simulink model "nb_107_32k_nb_fft_only_vlbi"  (R2018a) (continued)
== system nb_107_32k_nb_fft_only_vlbi == (continued)
      | port_label('input', 1, 'd');
      | port_label('output', 1, 'q');
      | disp(['Z^-', num2str(latency)]);
  pipeline22 : Subsystem  AncestorBlock=casper_library_delays/pipeline  mask(pipeline){latency=2}
      mask help:
      | An explicitly laid-out delay line to avoid mapping to SRL16s
      | for use in pipelining to help achieve timing closure.
      mask init:
      | pipeline_init(gcb,'latency',latency);
      settings: AttributesFormatString=Latency = %<latency>
      mask Display:
      | port_label('input', 1, 'd');
      | port_label('output', 1, 'q');
      | disp(['Z^-', num2str(latency)]);
  pipeline223 : Subsystem  Commented=on  AncestorBlock=casper_library_delays/pipeline  mask(pipeline){latency=5}
      mask help:
      | An explicitly laid-out delay line to avoid mapping to SRL16s
      | for use in pipelining to help achieve timing closure.
      mask init:
      | pipeline_init(gcb,'latency',latency);
      settings: AttributesFormatString=Latency = %<latency>
      mask Display:
      | port_label('input', 1, 'd');
      | port_label('output', 1, 'q');
      | disp(['Z^-', num2str(latency)]);
  pipeline23 : Subsystem  AncestorBlock=casper_library_delays/pipeline  mask(pipeline){csp_latency=2}
      mask prompts: csp_latency "Latency"
      mask help:
      | An explicitly laid-out delay line to avoid mapping to SRL16s
      | for use in pipelining to help achieve timing closure.
      mask init:
      | pipeline_init(gcb,'latency',csp_latency);
      settings: AttributesFormatString=Latency = %<latency>
      mask Display:
      | port_label('input', 1, 'd');
      | port_label('output', 1, 'q');
      | disp(['Z^-', num2str(csp_latency)]);
  pipeline234 : Subsystem  Commented=on  AncestorBlock=casper_library_delays/pipeline  mask(pipeline){latency=2}
      mask help:
      | An explicitly laid-out delay line to avoid mapping to SRL16s
      | for use in pipelining to help achieve timing closure.
      mask init:
      | pipeline_init(gcb,'latency',latency);
      settings: AttributesFormatString=Latency = %<latency>
      mask Display:
      | port_label('input', 1, 'd');
      | port_label('output', 1, 'q');
      | disp(['Z^-', num2str(latency)]);
  pipeline24 : Subsystem  AncestorBlock=casper_library_delays/pipeline  mask(pipeline){csp_latency=2}
      mask prompts: csp_latency "Latency"
      mask help:
      | An explicitly laid-out delay line to avoid mapping to SRL16s
      | for use in pipelining to help achieve timing closure.
      mask init:
      | pipeline_init(gcb,'latency',csp_latency);
      settings: AttributesFormatString=Latency = %<latency>
      mask Display:
      | port_label('input', 1, 'd');
      | port_label('output', 1, 'q');
      | disp(['Z^-', num2str(csp_latency)]);
  pipeline25 : Subsystem  AncestorBlock=casper_library_delays/pipeline  mask(pipeline){csp_latency=2}
      mask prompts: csp_latency "Latency"
      mask help:
      | An explicitly laid-out delay line to avoid mapping to SRL16s
      | for use in pipelining to help achieve timing closure.
      mask init:
      | pipeline_init(gcb,'latency',csp_latency);
      settings: AttributesFormatString=Latency = %<latency>
      mask Display:
      | port_label('input', 1, 'd');
      | port_label('output', 1, 'q');
      | disp(['Z^-', num2str(csp_latency)]);
  pipeline26 : Subsystem  AncestorBlock=casper_library_delays/pipeline  mask(pipeline){csp_latency=2}
      mask prompts: csp_latency "Latency"
      mask help:
      | An explicitly laid-out delay line to avoid mapping to SRL16s
      | for use in pipelining to help achieve timing closure.
      mask init:
      | pipeline_init(gcb,'latency',csp_latency);
      settings: AttributesFormatString=Latency = %<latency>
      mask Display:
      | port_label('input', 1, 'd');
      | port_label('output', 1, 'q');
      | disp(['Z^-', num2str(csp_latency)]);
  pipeline27 : Subsystem  AncestorBlock=casper_library_delays/pipeline  mask(pipeline){csp_latency=2}
      mask prompts: csp_latency "Latency"
      mask help:
      | An explicitly laid-out delay line to avoid mapping to SRL16s
      | for use in pipelining to help achieve timing closure.
      mask init:
      | pipeline_init(gcb,'latency',csp_latency);
      settings: AttributesFormatString=Latency = %<latency>
      mask Display:
      | port_label('input', 1, 'd');
      | port_label('output', 1, 'q');
      | disp(['Z^-', num2str(csp_latency)]);
  pipeline28 : Subsystem  AncestorBlock=casper_library_delays/pipeline  mask(pipeline){csp_latency=2}
      mask prompts: csp_latency "Latency"
      mask help:
      | An explicitly laid-out delay line to avoid mapping to SRL16s
      | for use in pipelining to help achieve timing closure.
      mask init:
      | pipeline_init(gcb,'latency',csp_latency);
      settings: AttributesFormatString=Latency = %<latency>
      mask Display:
      | port_label('input', 1, 'd');
      | port_label('output', 1, 'q');
      | disp(['Z^-', num2str(csp_latency)]);
  pipeline31 : Subsystem  AncestorBlock=casper_library_delays/pipeline  mask(pipeline){latency=2}
      mask help:
      | An explicitly laid-out delay line to avoid mapping to SRL16s
      | for use in pipelining to help achieve timing closure.
      mask init:
      | pipeline_init(gcb,'latency',latency);
      settings: AttributesFormatString=Latency = %<latency>
      mask Display:
      | port_label('input', 1, 'd');
      | port_label('output', 1, 'q');
      | disp(['Z^-', num2str(latency)]);
  pipeline38 : Subsystem  AncestorBlock=casper_library_delays/pipeline  mask(pipeline){csp_latency=2}
      mask prompts: csp_latency "Latency"
      mask help:
      | An explicitly laid-out delay line to avoid mapping to SRL16s
      | for use in pipelining to help achieve timing closure.
      mask init:
      | pipeline_init(gcb,'latency',csp_latency);
      settings: AttributesFormatString=Latency = %<latency>
      mask Display:
      | port_label('input', 1, 'd');
      | port_label('output', 1, 'q');
      | disp(['Z^-', num2str(csp_latency)]);
  pipeline39 : Subsystem  AncestorBlock=casper_library_delays/pipeline  mask(pipeline){csp_latency=2}
      mask prompts: csp_latency "Latency"
      mask help:
      | An explicitly laid-out delay line to avoid mapping to SRL16s
      | for use in pipelining to help achieve timing closure.
      mask init:
      | pipeline_init(gcb,'latency',csp_latency);
      settings: AttributesFormatString=Latency = %<latency>
      mask Display:
      | port_label('input', 1, 'd');
      | port_label('output', 1, 'q');
      | disp(['Z^-', num2str(csp_latency)]);
  pipeline4 : Subsystem  Commented=on  AncestorBlock=casper_library_delays/pipeline  mask(pipeline){latency=5}
      mask help:
      | An explicitly laid-out delay line to avoid mapping to SRL16s
      | for use in pipelining to help achieve timing closure.
      mask init:
      | pipeline_init(gcb,'latency',latency);
      settings: AttributesFormatString=Latency = %<latency>
      mask Display:
      | port_label('input', 1, 'd');
      | port_label('output', 1, 'q');
      | disp(['Z^-', num2str(latency)]);
  pipeline43 : Subsystem  AncestorBlock=casper_library_delays/pipeline  mask(pipeline){csp_latency=2}
      mask prompts: csp_latency "Latency"
      mask help:
      | An explicitly laid-out delay line to avoid mapping to SRL16s
      | for use in pipelining to help achieve timing closure.
      mask init:
      | pipeline_init(gcb,'latency',csp_latency);
      settings: AttributesFormatString=Latency = %<latency>
      mask Display:
      | port_label('input', 1, 'd');
      | port_label('output', 1, 'q');
      | disp(['Z^-', num2str(csp_latency)]);
  pipeline46 : Subsystem  Tag=cmac_delinked  mask(pipeline){csp_latency=2}
      mask prompts: csp_latency "Latency"
      mask help:
      | An explicitly laid-out delay line to avoid mapping to SRL16s
      | for use in pipelining to help achieve timing closure.
      mask init:
      | pipeline_init(gcb,'latency',csp_latency);
      settings: AttributesFormatString=Latency = %<latency>
      mask Display:
      | port_label('input', 1, 'd');
      | port_label('output', 1, 'q');
      | disp(['Z^-', num2str(csp_latency)]);
  pipeline47 : Subsystem  AncestorBlock=casper_library_delays/pipeline  mask(pipeline){csp_latency=2}
      mask prompts: csp_latency "Latency"
      mask help:
      | An explicitly laid-out delay line to avoid mapping to SRL16s
      | for use in pipelining to help achieve timing closure.
      mask init:
      | pipeline_init(gcb,'latency',csp_latency);
      settings: AttributesFormatString=Latency = %<latency>
      mask Display:
      | port_label('input', 1, 'd');
      | port_label('output', 1, 'q');
      | disp(['Z^-', num2str(csp_latency)]);
  pipeline5 : Subsystem  Commented=on  AncestorBlock=casper_library_delays/pipeline  mask(pipeline){latency=5}
      mask help:
      | An explicitly laid-out delay line to avoid mapping to SRL16s
      | for use in pipelining to help achieve timing closure.
      mask init:
      | pipeline_init(gcb,'latency',latency);
      settings: AttributesFormatString=Latency = %<latency>
      mask Display:
      | port_label('input', 1, 'd');
      | port_label('output', 1, 'q');
      | disp(['Z^-', num2str(latency)]);
  pipeline51 : Subsystem  AncestorBlock=casper_library_delays/pipeline  mask(pipeline){latency=2}
      mask help:
      | An explicitly laid-out delay line to avoid mapping to SRL16s
      | for use in pipelining to help achieve timing closure.
      mask init:
      | pipeline_init(gcb,'latency',latency);
      settings: AttributesFormatString=Latency = %<latency>
      mask Display:
      | port_label('input', 1, 'd');
      | port_label('output', 1, 'q');
      | disp(['Z^-', num2str(latency)]);
  pipeline52 : Subsystem  AncestorBlock=casper_library_delays/pipeline  mask(pipeline){csp_latency=2}
      mask prompts: csp_latency "Latency"
      mask help:
      | An explicitly laid-out delay line to avoid mapping to SRL16s
      | for use in pipelining to help achieve timing closure.
      mask init:
      | pipeline_init(gcb,'latency',csp_latency);
      settings: AttributesFormatString=Latency = %<latency>
      mask Display:
      | port_label('input', 1, 'd');
      | port_label('output', 1, 'q');
      | disp(['Z^-', num2str(csp_latency)]);
  pipeline59 : Subsystem  AncestorBlock=casper_library_delays/pipeline  mask(pipeline){csp_latency=2}
      mask prompts: csp_latency "Latency"
      mask help:
      | An explicitly laid-out delay line to avoid mapping to SRL16s
      | for use in pipelining to help achieve timing closure.
      mask init:
      | pipeline_init(gcb,'latency',csp_latency);
      settings: AttributesFormatString=Latency = %<latency>
      mask Display:
      | port_label('input', 1, 'd');
      | port_label('output', 1, 'q');
      | disp(['Z^-', num2str(csp_latency)]);
  pipeline6 : Subsystem  Commented=on  AncestorBlock=casper_library_delays/pipeline  mask(pipeline){latency=5}
      mask help:
      | An explicitly laid-out delay line to avoid mapping to SRL16s
      | for use in pipelining to help achieve timing closure.
      mask init:
      | pipeline_init(gcb,'latency',latency);
      settings: AttributesFormatString=Latency = %<latency>
      mask Display:
      | port_label('input', 1, 'd');
      | port_label('output', 1, 'q');
      | disp(['Z^-', num2str(latency)]);
  pipeline62 : Subsystem  AncestorBlock=casper_library_delays/pipeline  mask(pipeline){latency=2}
      mask help:
      | An explicitly laid-out delay line to avoid mapping to SRL16s
      | for use in pipelining to help achieve timing closure.
      mask init:
      | pipeline_init(gcb,'latency',latency);
      settings: AttributesFormatString=Latency = %<latency>
      mask Display:
      | port_label('input', 1, 'd');
      | port_label('output', 1, 'q');
      | disp(['Z^-', num2str(latency)]);
  pipeline66 : Subsystem  AncestorBlock=casper_library_delays/pipeline  mask(pipeline){csp_latency=2}
      mask prompts: csp_latency "Latency"
      mask help:
      | An explicitly laid-out delay line to avoid mapping to SRL16s
      | for use in pipelining to help achieve timing closure.
      mask init:
      | pipeline_init(gcb,'latency',csp_latency);
      settings: AttributesFormatString=Latency = %<latency>
      mask Display:
      | port_label('input', 1, 'd');
      | port_label('output', 1, 'q');
      | disp(['Z^-', num2str(csp_latency)]);
  pipeline7 : Subsystem  Commented=on  AncestorBlock=casper_library_delays/pipeline  mask(pipeline){latency=5}
      mask help:
      | An explicitly laid-out delay line to avoid mapping to SRL16s
      | for use in pipelining to help achieve timing closure.
      mask init:
      | pipeline_init(gcb,'latency',latency);
      settings: AttributesFormatString=Latency = %<latency>
      mask Display:
      | port_label('input', 1, 'd');
      | port_label('output', 1, 'q');
      | disp(['Z^-', num2str(latency)]);
  pipeline8 : Subsystem  AncestorBlock=casper_library_delays/pipeline  mask(pipeline){csp_latency=2}
      mask prompts: csp_latency "Latency"
      mask help:
      | An explicitly laid-out delay line to avoid mapping to SRL16s
      | for use in pipelining to help achieve timing closure.
      mask init:
      | pipeline_init(gcb,'latency',csp_latency);
      settings: AttributesFormatString=Latency = %<latency>
      mask Display:
      | port_label('input', 1, 'd');
      | port_label('output', 1, 'q');
      | disp(['Z^-', num2str(csp_latency)]);
  pipeline9 : Subsystem  Commented=on  AncestorBlock=casper_library_delays/pipeline  mask(pipeline){latency=5}
      mask help:
      | An explicitly laid-out delay line to avoid mapping to SRL16s
      | for use in pipelining to help achieve timing closure.
      mask init:
      | pipeline_init(gcb,'latency',latency);
      settings: AttributesFormatString=Latency = %<latency>
      mask Display:
      | port_label('input', 1, 'd');
      | port_label('output', 1, 'q');
      | disp(['Z^-', num2str(latency)]);
  pipeline93 : Subsystem  AncestorBlock=casper_library_delays/pipeline  mask(pipeline){csp_latency=2}
      mask prompts: csp_latency "Latency"
      mask help:
      | An explicitly laid-out delay line to avoid mapping to SRL16s
      | for use in pipelining to help achieve timing closure.
      mask init:
      | pipeline_init(gcb,'latency',csp_latency);
      settings: AttributesFormatString=Latency = %<latency>
      mask Display:
      | port_label('input', 1, 'd');
      | port_label('output', 1, 'q');
      | disp(['Z^-', num2str(csp_latency)]);
  pipeline96 : Subsystem  AncestorBlock=casper_library_delays/pipeline  mask(pipeline){latency=2}
      mask help:
      | An explicitly laid-out delay line to avoid mapping to SRL16s
      | for use in pipelining to help achieve timing closure.
      mask init:
      | pipeline_init(gcb,'latency',latency);
      settings: AttributesFormatString=Latency = %<latency>
      mask Display:
      | port_label('input', 1, 'd');
      | port_label('output', 1, 'q');
      | disp(['Z^-', num2str(latency)]);
  pipeline97 : Subsystem  AncestorBlock=casper_library_delays/pipeline  mask(pipeline){latency=2}
      mask help:
      | An explicitly laid-out delay line to avoid mapping to SRL16s
      | for use in pipelining to help achieve timing closure.
      mask init:
      | pipeline_init(gcb,'latency',latency);
      settings: AttributesFormatString=Latency = %<latency>
      mask Display:
      | port_label('input', 1, 'd');
      | port_label('output', 1, 'q');
      | disp(['Z^-', num2str(latency)]);
  pipeline98 : Subsystem  AncestorBlock=casper_library_delays/pipeline  mask(pipeline){latency=2}
      mask help:
      | An explicitly laid-out delay line to avoid mapping to SRL16s
      | for use in pipelining to help achieve timing closure.
      mask init:
      | pipeline_init(gcb,'latency',latency);
      settings: AttributesFormatString=Latency = %<latency>
      mask Display:
      | port_label('input', 1, 'd');
      | port_label('output', 1, 'q');
      | disp(['Z^-', num2str(latency)]);
  pipeline99 : Subsystem  AncestorBlock=casper_library_delays/pipeline  mask(pipeline){latency=2}
      mask help:
      | An explicitly laid-out delay line to avoid mapping to SRL16s
      | for use in pipelining to help achieve timing closure.
      mask init:
      | pipeline_init(gcb,'latency',latency);
      settings: AttributesFormatString=Latency = %<latency>
      mask Display:
      | port_label('input', 1, 'd');
      | port_label('output', 1, 'q');
      | disp(['Z^-', num2str(latency)]);
  pkt_len : Subsystem  Tag=xps:sw_reg  AncestorBlock=xps_library/Memory/software_register  mask(swreg){io_dir=From Processor, io_delay=1, init_val=2^9-1, sample_period=1, names=len, bitwidths=9, bin_pts=0, arith_types=0, sim_port=on, show_format=on}
      mask prompts: io_dir "I/O direction"; io_delay "I/O delay"; init_val "Initial Value"; sample_period "Sample period"; names "Bitfield names [msb...lsb]"; bitwidths "Bitfield widths"; bin_pts "Bitfield binary pts"; arith_types "Bitfield types, ufix=0, fix=1, bool=2"; sim_port "Provide sim input/output?"; show_format "Print format string?"
      mask help: A 32-bit software-accessible register. Can be divided into bitfields of varying widths and types using the fields in the block mask.
      mask init:
      | swreg_init(gcb)
      settings: AttributesFormatString=1input:uf9.0 = 9 bits
      mask TypeOptions io_dir: To Processor | From Processor
      mask Callback io_dir:
      | mask_enables = get_param(gcb, 'MaskEnables');
      | mask_names = get_param(gcb, 'MaskNames');
      | if strcmp(get_param(gcb, 'io_dir'), 'To Processor'),
      |     mask_enables{ismember(mask_names, 'sample_period')} = 'off';
      | else
      |     mask_enables{ismember(mask_names, 'sample_period')} = 'on';
      | end
      | set_param(gcb, 'MaskEnables', mask_enables);
      mask DialogControl ParameterTabVar0 Prompt: Setup
  pkt_len_sel : Subsystem  Tag=xps:sw_reg  AncestorBlock=xps_library/Memory/software_register  mask(swreg){io_dir=From Processor, io_delay=1, init_val=0, sample_period=1, names=sel, bitwidths=1, bin_pts=0, arith_types=2, sim_port=on, show_format=on}
      mask prompts: io_dir "I/O direction"; io_delay "I/O delay"; init_val "Initial Value"; sample_period "Sample period"; names "Bitfield names [msb...lsb]"; bitwidths "Bitfield widths"; bin_pts "Bitfield binary pts"; arith_types "Bitfield types, ufix=0, fix=1, bool=2"; sim_port "Provide sim input/output?"; show_format "Print format string?"
      mask help: A 32-bit software-accessible register. Can be divided into bitfields of varying widths and types using the fields in the block mask.
      mask init:
      | swreg_init(gcb)
      settings: AttributesFormatString=1input:b = 1 bits
      mask TypeOptions io_dir: To Processor | From Processor
      mask Callback io_dir:
      | mask_enables = get_param(gcb, 'MaskEnables');
      | mask_names = get_param(gcb, 'MaskNames');
      | if strcmp(get_param(gcb, 'io_dir'), 'To Processor'),
      |     mask_enables{ismember(mask_names, 'sample_period')} = 'off';
      | else
      |     mask_enables{ismember(mask_names, 'sample_period')} = 'on';
      | end
      | set_param(gcb, 'MaskEnables', mask_enables);
      mask DialogControl ParameterTabVar0 Prompt: Setup
  re_im_interleave : Subsystem
  rx_dest_ip : Subsystem  Tag=xps:sw_reg  AncestorBlock=xps_library/software register  mask(swreg){io_dir=To Processor, io_delay=0, sample_period=1, names=reg, bitwidths=32, bin_pts=0, arith_types=0, sim_port=off, show_format=on}
      mask prompts: io_dir "I/O direction"; io_delay "I/O delay"; sample_period "Sample period"; names "Bitfield names [msb...lsb]"; bitwidths "Bitfield widths"; bin_pts "Bitfield binary pts"; arith_types "Bitfield types, ufix=0, fix=1, bool=2"; sim_port "Provide sim input/output?"; show_format "Print format string?"
      mask help: A 32-bit software-accessible register. Can be divided into bitfields of varying widths and types using the fields in the block mask.
      mask init:
      | swreg_init(gcb)
      settings: AttributesFormatString=1output:uf32.0 = 32 bits
      mask TypeOptions io_dir: To Processor | From Processor
      mask Callback io_dir:
      | mask_enables = get_param(gcb, 'MaskEnables');
      | mask_names = get_param(gcb, 'MaskNames');
      | if strcmp(get_param(gcb, 'io_dir'), 'To Processor'),
      |     mask_enables{ismember(mask_names, 'sample_period')} = 'off';
      | else
      |     mask_enables{ismember(mask_names, 'sample_period')} = 'on';
      | end
      | set_param(gcb, 'MaskEnables', mask_enables);
  scale_wng0 : Subsystem  Tag=xps:sw_reg  AncestorBlock=xps_library/Memory/software_register  mask(swreg){io_dir=From Processor, io_delay=1, init_val=0, sample_period=1, names=scale, bitwidths=16, bin_pts=15, arith_types=0, sim_port=on, show_format=on}
      mask prompts: io_dir "I/O direction"; io_delay "I/O delay"; init_val "Initial Value"; sample_period "Sample period"; names "Bitfield names [msb...lsb]"; bitwidths "Bitfield widths"; bin_pts "Bitfield binary pts"; arith_types "Bitfield types, ufix=0, fix=1, bool=2"; sim_port "Provide sim input/output?"; show_format "Print format string?"
      mask help: A 32-bit software-accessible register. Can be divided into bitfields of varying widths and types using the fields in the block mask.
      mask init:
      | swreg_init(gcb)
      settings: AttributesFormatString=1input:uf16.15 = 16 bits
      mask TypeOptions io_dir: To Processor | From Processor
      mask Callback io_dir:
      | mask_enables = get_param(gcb, 'MaskEnables');
      | mask_names = get_param(gcb, 'MaskNames');
      | if strcmp(get_param(gcb, 'io_dir'), 'To Processor'),
      |     mask_enables{ismember(mask_names, 'sample_period')} = 'off';
      | else
      |     mask_enables{ismember(mask_names, 'sample_period')} = 'on';
      | end
      | set_param(gcb, 'MaskEnables', mask_enables);
      mask DialogControl ParameterTabVar0 Prompt: Setup
  snap_cwg : Subsystem  Tag=casper:bitsnap  Commented=on  AncestorBlock=casper_library_scopes/bitfield_snapshot  mask(bitsnap){io_names=[p0_d0 p0_d1 p0_d2 p0_d3 p0_d4 p0_d5 p0_d6 p0_d7 dv sync], io_widths=[10 10 10 10 10 10 10 10 1 1], io_bps=[9 9 9 9 9 9 9 9 0 0], io_types=[1 1 1 1 1 1 1 1 2 2], show_format=on, snap_storage=bram, snap_dram_dimm=2, snap_dram_clock=250, snap_nsamples=10, snap_data_width=128, snap_offset=off, snap_circap=off, snap_value=off, snap_ext_arm=off, snap_ext_circ=off, snap_provide_outputs=on, snap_use_dsp48=on, snap_delay=2, extra_names=timestamp, extra_widths=32, extra_bps=0, extra_types=0}
      mask prompts: io_names "Names [msb...lsb]"; io_widths "Bitwidths [msb...lsb]"; io_bps "Binary pts [msb...lsb]"; io_types "Data types [msb...lsb], ufix=0, fix=1, bool=2"; show_format "Print format string?"; snap_storage "Storage medium"; snap_dram_dimm "DRAM dimm"; snap_dram_clock "DRAM clock rate"; snap_nsamples "Number of Samples (2^?)"; snap_data_width "Data width"; snap_offset "Start delay support"; snap_circap "Circular capture support"; snap_value "Extra value capture support"; snap_ext_arm "External arm support"; snap_ext_circ "External circular capture support"; snap_provide_outputs "Provide outputs"; snap_use_dsp48 "Use DSP48s to implement counters"; snap_delay "Pre-snapshot delay"; extra_names "Names [msb...lsb]"; extra_widths "Widths [msb...lsb]"; extra_bps "Binary pts [msb...lsb]"; extra_types "Data types [msb...lsb], ufix=0, fix=1, bool=2"
      mask help:
      | A wrapped snapshot block. Allows specification of inputs to a snapblock word so the toolflow can automatically set up the scope.
      | See documentation for snapshot for explanation of parameters.
      mask init:
      | bitsnap_init(gcb);
      settings: AttributesFormatString=|
      | snap(10):f10.9,f10.9,f10.9,f10.9,f10.9,f10.9,f10.9,f10.9,b,b = 82 bits\n128 wide, 1024 deep
      | debugID: 22238404
      mask TypeOptions snap_storage: bram | dram
      mask Callback snap_storage: bitsnap_callback();
      mask TypeOptions snap_dram_dimm: 1 | 2 | 3 | 4
      mask TypeOptions snap_dram_clock: 200 | 250 | 266 | 300 | 333
      mask TypeOptions snap_data_width: 8 | 16 | 32 | 64 | 128
      mask Callback snap_value: bitsnap_callback();
      mask Callback snap_ext_arm: bitsnap_callback();
      mask Callback snap_ext_circ: bitsnap_callback();
      mask Callback snap_provide_outputs: bitsnap_callback();
      mask DialogControl ParameterTabVar0 Prompt: Inputs
      mask DialogControl ParameterTabVar01 Prompt: Snapshot
      mask DialogControl ParameterTabVar012 Prompt: Extra Value
  snap_dds : Subsystem  Tag=casper:bitsnap  Commented=on  AncestorBlock=casper_library_scopes/bitfield_snapshot  mask(bitsnap){io_names=[p0_d0 p0_d1 p0_d2 p0_d3 dv sync], io_widths=[18 18 18 18 1 1], io_bps=[17 17 17 17 0 0], io_types=[1 1 1 1 2 2], show_format=on, snap_storage=bram, snap_dram_dimm=2, snap_dram_clock=250, snap_nsamples=10, snap_data_width=128, snap_offset=off, snap_circap=off, snap_value=off, snap_ext_arm=off, snap_ext_circ=off, snap_provide_outputs=on, snap_use_dsp48=on, snap_delay=2, extra_names=timestamp, extra_widths=32, extra_bps=0, extra_types=0}
      mask prompts: io_names "Names [msb...lsb]"; io_widths "Bitwidths [msb...lsb]"; io_bps "Binary pts [msb...lsb]"; io_types "Data types [msb...lsb], ufix=0, fix=1, bool=2"; show_format "Print format string?"; snap_storage "Storage medium"; snap_dram_dimm "DRAM dimm"; snap_dram_clock "DRAM clock rate"; snap_nsamples "Number of Samples (2^?)"; snap_data_width "Data width"; snap_offset "Start delay support"; snap_circap "Circular capture support"; snap_value "Extra value capture support"; snap_ext_arm "External arm support"; snap_ext_circ "External circular capture support"; snap_provide_outputs "Provide outputs"; snap_use_dsp48 "Use DSP48s to implement counters"; snap_delay "Pre-snapshot delay"; extra_names "Names [msb...lsb]"; extra_widths "Widths [msb...lsb]"; extra_bps "Binary pts [msb...lsb]"; extra_types "Data types [msb...lsb], ufix=0, fix=1, bool=2"
      mask help:
      | A wrapped snapshot block. Allows specification of inputs to a snapblock word so the toolflow can automatically set up the scope.
      | See documentation for snapshot for explanation of parameters.
      mask init:
      | bitsnap_init(gcb);
      settings: AttributesFormatString=|
      | snap(6):f18.17,f18.17,f18.17,f18.17,b,b = 74 bits\n128 wide, 1024 deep
      | debugID: 33678148
      mask TypeOptions snap_storage: bram | dram
      mask Callback snap_storage: bitsnap_callback();
      mask TypeOptions snap_dram_dimm: 1 | 2 | 3 | 4
      mask TypeOptions snap_dram_clock: 200 | 250 | 266 | 300 | 333
      mask TypeOptions snap_data_width: 8 | 16 | 32 | 64 | 128
      mask Callback snap_value: bitsnap_callback();
      mask Callback snap_ext_arm: bitsnap_callback();
      mask Callback snap_ext_circ: bitsnap_callback();
      mask Callback snap_provide_outputs: bitsnap_callback();
      mask DialogControl ParameterTabVar0 Prompt: Inputs
      mask DialogControl ParameterTabVar01 Prompt: Snapshot
      mask DialogControl ParameterTabVar012 Prompt: Extra Value
  snap_dds1 : Subsystem  Tag=casper:bitsnap  Commented=on  AncestorBlock=casper_library_scopes/bitfield_snapshot  mask(bitsnap){io_names=[p0_d0 p0_d1 p0_d2 p0_d3 dv sync], io_widths=[18 18 18 18 1 1], io_bps=[17 17 17 17 0 0], io_types=[1 1 1 1 2 2], show_format=on, snap_storage=bram, snap_dram_dimm=2, snap_dram_clock=250, snap_nsamples=10, snap_data_width=128, snap_offset=off, snap_circap=off, snap_value=off, snap_ext_arm=off, snap_ext_circ=off, snap_provide_outputs=on, snap_use_dsp48=on, snap_delay=2, extra_names=timestamp, extra_widths=32, extra_bps=0, extra_types=0}
      mask prompts: io_names "Names [msb...lsb]"; io_widths "Bitwidths [msb...lsb]"; io_bps "Binary pts [msb...lsb]"; io_types "Data types [msb...lsb], ufix=0, fix=1, bool=2"; show_format "Print format string?"; snap_storage "Storage medium"; snap_dram_dimm "DRAM dimm"; snap_dram_clock "DRAM clock rate"; snap_nsamples "Number of Samples (2^?)"; snap_data_width "Data width"; snap_offset "Start delay support"; snap_circap "Circular capture support"; snap_value "Extra value capture support"; snap_ext_arm "External arm support"; snap_ext_circ "External circular capture support"; snap_provide_outputs "Provide outputs"; snap_use_dsp48 "Use DSP48s to implement counters"; snap_delay "Pre-snapshot delay"; extra_names "Names [msb...lsb]"; extra_widths "Widths [msb...lsb]"; extra_bps "Binary pts [msb...lsb]"; extra_types "Data types [msb...lsb], ufix=0, fix=1, bool=2"
      mask help:
      | A wrapped snapshot block. Allows specification of inputs to a snapblock word so the toolflow can automatically set up the scope.
      | See documentation for snapshot for explanation of parameters.
      mask init:
      | bitsnap_init(gcb);
      settings: AttributesFormatString=|
      | snap(6):f18.17,f18.17,f18.17,f18.17,b,b = 74 bits\n128 wide, 1024 deep
      | debugID: 104492841
      mask TypeOptions snap_storage: bram | dram
      mask Callback snap_storage: bitsnap_callback();
      mask TypeOptions snap_dram_dimm: 1 | 2 | 3 | 4
      mask TypeOptions snap_dram_clock: 200 | 250 | 266 | 300 | 333
      mask TypeOptions snap_data_width: 8 | 16 | 32 | 64 | 128
      mask Callback snap_value: bitsnap_callback();
      mask Callback snap_ext_arm: bitsnap_callback();
      mask Callback snap_ext_circ: bitsnap_callback();
      mask Callback snap_provide_outputs: bitsnap_callback();
      mask DialogControl ParameterTabVar0 Prompt: Inputs
      mask DialogControl ParameterTabVar01 Prompt: Snapshot
      mask DialogControl ParameterTabVar012 Prompt: Extra Value
  wng0 : Subsystem  mask{seed1=hex2dec('12345678'), seed2=hex2dec('87654321'), seed3=hex2dec('45612378'), seed4=hex2dec('85274163')}
      mask prompts: seed1 "Seed 1"; seed2 "Seed 2"; seed3 "Seed 3"; seed4 "Seed 4"
  xsg_core_config : xps_library/Platforms/SKARAB  Tag=xps:xsg  hw_sys=SKARAB:xc7vx690t  clk_src=sys_clk  clk_rate=238  sample_period=1  synthesis_tool=XST
      settings: SourceProductName=CASPER XPS Blockset
  tag scope: cnt_rst=global, force_gbe_txen=global, local_time_capture=global, local_time_src=global, pfb_bypass=global, snap_reord_mux_we_sel=global, snap_stop_mux_sel=global, sys_rst=global, ts_tvg_en=global
  wires:
  Gateway Out20/1 -> To Workspace20/1
  DDC/1(ddc_sync) -> [ddc_sync]
  DDC/3(ddc_pol1) -> [ddc_pol1]
  DDC/4(ddc_dv) -> [ddc_dvalid]
  Gateway Out65/1 -> To Workspace69/1
  [ddc_dvalid] -> Gateway Out65/1
  [ddc_sync] -> Gateway Out21/1
  Gateway Out21/1 -> To Workspace23/1
  DDC/2(ddc_pol0) -> [ddc_pol0]
  Counter10/1 -> Relational5/1, Relational2/1
  Logical17/1 -> Gateway Out37/1, Register/2, Counter10/1
  [sys_rst] -> Logical17/1
  Relational5/1 -> Logical17/2
  Gateway Out19/1 -> To Workspace11/1
  fft_fwd_inv/1(in_fwd_inv) -> pipeline234/1(d)
  pipeline234/1(q) -> Goto115/1
  pipeline223/1(q) -> bus_expand5/1(bus_in)
  From15/1 -> pipeline2/1(d), pipeline216/1(d)
  pipeline216/1(q) -> snap_dds/5(in_dv)
  From16/1 -> pipeline13/1(d), pipeline217/1(d)
  pipeline217/1(q) -> snap_dds/6(in_sync)
  pipeline13/1(q) -> snap_dds/8(trig)
  pipeline11/1(q) -> bus_expand2/1(bus_in)
  From18/1 -> pipeline1/1(d), pipeline9/1(d)
  From21/1 -> pipeline11/1(d)
  bus_expand2/4(out5) -> snap_cwg/4(in_p0_d3)
  bus_expand2/5(out4) -> snap_cwg/5(in_p0_d4)
  bus_expand2/1(msb_out8) -> snap_cwg/1(in_p0_d0)
  bus_expand2/2(out7) -> snap_cwg/2(in_p0_d1)
  bus_expand2/3(out6) -> snap_cwg/3(in_p0_d2)
  bus_expand2/6(out3) -> snap_cwg/6(in_p0_d5)
  bus_expand2/7(out2) -> snap_cwg/7(in_p0_d6)
  bus_expand2/8(lsb_out1) -> snap_cwg/8(in_p0_d7)
  pipeline9/1(q) -> snap_cwg/9(in_dv)
  From20/1 -> pipeline12/1(d), pipeline10/1(d)
  pipeline10/1(q) -> snap_cwg/10(in_sync)
  pipeline12/1(q) -> snap_cwg/12(trig)
  Constant4/1 -> gap_offset/1(sim_1)
  Constant55/1 -> AddSub3/2
  AddSub3/1 -> Gateway Out39/1, Relational5/2
  gap_offset/1(in_offset) -> pipeline14/1(d)
  Constant21/1 -> forty_gbe/8(rx_overrun_ack)
  Constant20/1 -> forty_gbe/7(rx_ack)
  [gbe_rst] -> pipeline99/1(d)
  [gbe_cnt_rst] -> pipeline31/1(d)
  pipeline31/1(q) -> forty_gbe/9(debug_rst)
  forty_gbe/13(rx_dest_ip) -> pipeline115/1(d)
  forty_gbe/14(rx_dest_port) -> pipeline116/1(d)
  [rx_dest_ip] -> pipeline/1(d)
  pipeline/1(q) -> rx_dest_ip/1(out_reg)
  forty_gbe/1(led_up) -> pipeline100/1(d)
  [forty_gbe_led_up] -> pipeline20/1(d)
  forty_gbe/2(led_rx) -> pipeline101/1(d)
  [forty_gbe_led_rx] -> pipeline22/1(d)
  forty_gbe/3(led_tx) -> pipeline102/1(d)
  [forty_gbe_led_tx] -> pipeline51/1(d)
  forty_gbe/4(tx_afull) -> pipeline103/1(d)
  forty_gbe/5(tx_overflow) -> pipeline104/1(d)
  forty_gbe/12(rx_overrun) -> pipeline114/1(d)
  pipeline20/1(q) -> gbe_status/1(out_led_up)
  pipeline22/1(q) -> gbe_status/2(out_led_rx)
  pipeline51/1(q) -> gbe_status/3(out_led_tx)
  forty_gbe/8(rx_source_ip) -> pipeline110/1(d)
  forty_gbe/9(rx_source_port) -> pipeline111/1(d)
  forty_gbe/6(rx_data) -> pipeline108/1(d)
  forty_gbe/10(rx_end_of_frame) -> pipeline112/1(d)
  forty_gbe/11(rx_bad_frame) -> pipeline113/1(d)
  [s256_d] -> pipeline98/1(d)
  [s256_ip] -> pipeline97/1(d)
  [s256_port] -> pipeline96/1(d)
  Logical3/1 -> forty_gbe/6(tx_end_of_frame)
  [s256_v] -> Logical2/2
  [s256_eof] -> Logical3/2
  Delay4/1 -> Logical2/1, Logical3/1
  [s256_eof] -> Delay4/2
  pipeline96/1(q) -> forty_gbe/5(tx_dest_port)
  pipeline97/1(q) -> forty_gbe/4(tx_dest_ip)
  pipeline98/1(q) -> forty_gbe/2(tx_data)
  pipeline99/1(q) -> forty_gbe/1(rst)
  Logical5/1 -> Delay4/1
  pipeline100/1(q) -> [forty_gbe_led_up]
  pipeline101/1(q) -> [forty_gbe_led_rx]
  pipeline102/1(q) -> [forty_gbe_led_tx]
  pipeline103/1(q) -> [forty_gbe_tx_full]
  pipeline104/1(q) -> [forty_gbe_tx_of]
  pipeline108/1(q) -> [forty_gbe_rx_data]
  pipeline110/1(q) -> [forty_gbe_rx_src_ip]
  pipeline111/1(q) -> [forty_gbe_rx_src_port]
  pipeline112/1(q) -> [forty_gbe_rx_eof]
  pipeline113/1(q) -> [forty_gbe_rx_bad]
  pipeline114/1(q) -> [forty_gbe_rx_overrun]
  pipeline115/1(q) -> [rx_dest_ip]
  pipeline116/1(q) -> [rx_dest_port]
  Logical2/1 -> forty_gbe/3(tx_valid)
  forty_gbe/7(rx_valid) -> pipeline62/1(d)
  pipeline62/1(q) -> [forty_gbe_rx_valid]
  [gbe_txen] -> Logical5/2
  [force_gbe_txen] -> Logical7/2
  Logical7/1 -> Logical5/1
  edge_detect7/1(out) -> pipeline24/1(d)
  control/7(in_cnt_rst) -> edge_detect5/1(in)
  edge_detect5/1(out) -> pipeline18/1(d)
  control/3(in_gbe_rst) -> pipeline25/1(d)
  control/1(in_sys_rst) -> pipeline23/1(d)
  pipeline23/1(q) -> [sys_rst]
  pipeline24/1(q) -> [clr_status]
  pipeline25/1(q) -> [gbe_rst]
  pipeline26/1(q) -> [gbe_txen]
  pipeline17/1(q) -> [tvg_adc0_en]
  pipeline18/1(q) -> [cnt_rst]
  control/5(in_tvg_adc0) -> pipeline17/1(d)
  edge_detect6/1(out) -> pipeline38/1(d)
  pipeline38/1(q) -> [gbe_cnt_rst]
  control/8(in_gbe_cnt_rst) -> edge_detect6/1(in)
  control/4(in_gbe_txen) -> pipeline26/1(d)
  control/2(in_status_clr) -> edge_detect7/1(in)
  pipeline43/1(q) -> [adc_snap_arm]
  control/9(in_adc_snap_arm) -> pipeline43/1(d)
  control/10(in_adc_snap_trig_select) -> pipeline39/1(d)
  pipeline39/1(q) -> [adc_snap_trig_source]
  pipeline59/1(q) -> [ct_tvg_en]
  control/11(in_ct_tvg_en) -> pipeline59/1(d)
  pipeline52/1(q) -> [up_dbg_rst]
  control/12(in_unpack_debug_reset) -> pipeline52/1(d)
  control/13(in_cd_bypass) -> pipeline131/1(d)
  pipeline131/1(q) -> [cd_bypass]
  pipeline93/1(q) -> [tvg_adc1_en]
  control/6(in_tvg_adc1) -> pipeline93/1(d)
  control/14(in_gbe_stopmux) -> pipeline27/1(d)
  pipeline27/1(q) -> [gbe_stopmux]
  control/15(in_pfb_bypass) -> pipeline66/1(d)
  pipeline66/1(q) -> [pfb_bypass]
  control/16(in_ts_tvg_en) -> pipeline148/1(d)
  pipeline148/1(q) -> [ts_tvg_en]
  control/17(in_force_gbe_txen) -> pipeline21/1(d)
  pipeline21/1(q) -> [force_gbe_txen]
  control/18(in_snap_stop_mux_sel) -> pipeline127/1(d)
  control/19(in_snap_reord_mux_we_sel) -> pipeline128/1(d)
  pipeline127/1(q) -> [snap_stop_mux_sel]
  pipeline128/1(q) -> [snap_reord_mux_we_sel]
  control/20(in_local_time_source) -> pipeline16/1(d)
  edge_detect8/1(out) -> pipeline164/1(d)
  pipeline164/1(q) -> [local_time_capture]
  pipeline16/1(q) -> [local_time_src]
  control/21(in_local_time_capture) -> edge_detect8/1(in)
  control/22(in_time_diff_check_en) -> pipeline47/1(d)
  pipeline47/1(q) -> [time_diff_check_en]
  control/23(in_auto_rst_enable) -> pipeline132/1(d)
  pipeline132/1(q) -> [auto_rst_enable]
  pipeline155/1(q) -> [pipeline_stall]
  control/24(in_pipeline_stall) -> pipeline155/1(d)
  [board_in_fault] -> Inverter/1
  Inverter/1 -> Logical7/1
  Constant18/1 -> [board_in_fault]
  Constant22/1 -> [s256_d]
  Constant23/1 -> [s256_v]
  Constant24/1 -> [s256_eof]
  Constant25/1 -> [s256_port]
  Constant26/1 -> [s256_ip]
  Constant27/1 -> fft_fwd_inv/1(sim_1)
  fft_shift/1(in_fft_shift) -> fft_shift_munge/1(din)
  pipeline15/1(q) -> [fft_shift]
  pipeline1/1(q) -> snap_cwg/11(we)
  pipeline2/1(q) -> snap_dds/7(we)
  [ddc_sync_in] -> DDC/1(sync)
  [ddc_dv_in] -> DDC/2(dvalid)
  [osc_cmplx] -> DDC/6(osc1), DDC/4(osc0)
  [osc_temp_dv] -> Delay3/1
  cwg_cos/2(data_out) -> Delay8/1
  [osc_freq] -> dds_freq_idx_offset_calc/1(ddc_freq)
  dds_freq_idx_offset_calc/1(dds_fftbin_offset) -> [dds_fft_phase_offset]
  [osc_freq] -> cwg_cos/2(freq)
  freq_cwg_osc/1(in_frequency) -> [osc_freq]
  Gateway Out10/1 -> To Workspace12/1
  Gateway Out1/1 -> To Workspace10/1
  bus_expand3/1(msb_out8) -> Gateway Out10/1
  bus_expand3/2(out7) -> Gateway Out1/1
  bus_expand3/3(out6) -> Gateway Out24/1
  bus_expand3/4(out5) -> Gateway Out25/1
  bus_expand3/5(out4) -> Gateway Out26/1
  bus_expand3/6(out3) -> Gateway Out27/1
  bus_expand3/7(out2) -> Gateway Out28/1
  bus_expand3/8(lsb_out1) -> Gateway Out29/1
  [osc_cos_ddc0] -> re_im_interleave/1(In1)
  [osc_sin_ddc0] -> re_im_interleave/2(In2)
  re_im_interleave/1(Out1) -> [osc_cmplx]
  From1/1 -> bus_expand3/1(bus_in)
  Gateway Out24/1 -> To Workspace24/1
  Gateway Out25/1 -> To Workspace25/1
  Gateway Out26/1 -> To Workspace26/1
  Gateway Out27/1 -> To Workspace27/1
  Gateway Out28/1 -> To Workspace28/1
  Gateway Out29/1 -> To Workspace29/1
  bus_expand4/1(msb_out2) -> Gateway Out22/1
  Gateway Out22/1 -> To Workspace22/1
  bus_expand4/2(lsb_out1) -> Gateway Out23/1
  Gateway Out23/1 -> To Workspace1/1
  [ddc_pol0] -> bus_expand4/1(bus_in)
  [ddc_pol0_in] -> DDC/5(pol1_data), DDC/3(pol0_data)
  [cwg_awgn] -> complexify/1(In1)
  complexify/1(Out1) -> [cwg_cmplx]
  freq_cwg_input/1(in_frequency) -> Gateway Out34/1, cwg2/2(freq)
  [sys_rst] -> cwg2/4(rst)
  [sys_rst] -> Register2/2
  edge_detect2/1(out) -> Gateway Out19/1, cwg2/1(sync)
  Delay2/1 -> Gateway Out20/1, cwg2/3(dv)
  Gateway Out30/1 -> To Workspace30/1
  Gateway Out31/1 -> To Workspace31/1
  Delay3/1 -> cwg_cos/3(dv)
  [sys_rst] -> cwg_cos/4(rst)
  Constant3/1 -> cwg_cos/1(sync)
  cwg_sin/2(data_out) -> Delay9/1
  [sys_rst] -> cwg_sin/4(rst)
  Delay5/1 -> cwg_sin/3(dv)
  [osc_temp_dv] -> Delay5/1
  [osc_freq] -> cwg_sin/2(freq)
  Constant5/1 -> cwg_sin/1(sync)
  Gateway Out32/1 -> To Workspace32/1
  [ddc_of] -> Gateway Out32/1
  DDC/5(ddc_of) -> [ddc_of]
  fft_shift_munge/1(dout) -> pipeline15/1(d)
  cwg_scale/1(in_scale) -> Gateway Out33/1, cwg2/5(scale)
  Constant7/1 -> cwg_cos/5(scale)
  Constant15/1 -> cwg_sin/5(scale)
  Constant16/1 -> cwg_scale/1(sim_1)
  Gateway Out33/1 -> To Workspace33/1
  Gateway Out3/1 -> To Workspace4/1
  bus_expand18/1(msb_out8) -> Gateway Out3/1
  Gateway Out2/1 -> To Workspace2/1
  bus_expand18/2(out7) -> Gateway Out2/1
  Gateway Out4/1 -> To Workspace3/1
  bus_expand18/3(out6) -> Gateway Out4/1
  Gateway Out5/1 -> To Workspace5/1
  bus_expand18/4(out5) -> Gateway Out5/1
  Gateway Out6/1 -> To Workspace6/1
  bus_expand18/5(out4) -> Gateway Out6/1
  Gateway Out7/1 -> To Workspace7/1
  bus_expand18/6(out3) -> Gateway Out7/1
  Gateway Out8/1 -> To Workspace8/1
  bus_expand18/7(out2) -> Gateway Out8/1
  Gateway Out9/1 -> To Workspace9/1
  bus_expand18/8(lsb_out1) -> Gateway Out9/1
  From/1 -> bus_expand18/1(bus_in)
  Gateway Out12/1 -> To Workspace13/1
  Gateway Out11/1 -> To Workspace14/1
  Gateway Out13/1 -> To Workspace15/1
  Gateway Out14/1 -> To Workspace16/1
  Gateway Out15/1 -> To Workspace17/1
  Gateway Out16/1 -> To Workspace18/1
  Gateway Out17/1 -> To Workspace19/1
  Gateway Out18/1 -> To Workspace21/1
  bus_expand1/1(msb_out8) -> Gateway Out12/1
  bus_expand1/2(out7) -> Gateway Out11/1
  bus_expand1/3(out6) -> Gateway Out13/1
  bus_expand1/4(out5) -> Gateway Out14/1
  bus_expand1/5(out4) -> Gateway Out15/1
  bus_expand1/6(out3) -> Gateway Out16/1
  bus_expand1/7(out2) -> Gateway Out17/1
  bus_expand1/8(lsb_out1) -> Gateway Out18/1
  From12/1 -> bus_expand1/1(bus_in)
  Register2/1 -> Delay1/1
  From41/1 -> pipeline223/1(d)
  From42/1 -> pipeline6/1(d), pipeline5/1(d)
  pipeline6/1(q) -> snap_dds1/5(in_dv)
  From43/1 -> pipeline7/1(d), pipeline4/1(d)
  pipeline7/1(q) -> snap_dds1/6(in_sync)
  pipeline4/1(q) -> snap_dds1/8(trig)
  pipeline5/1(q) -> snap_dds1/7(we)
  bus_expand5/1(msb_out8) -> snap_dds/1(in_p0_d0)
  bus_expand5/2(out7) -> snap_dds/2(in_p0_d1)
  bus_expand5/3(out6) -> snap_dds/3(in_p0_d2)
  bus_expand5/4(out5) -> snap_dds/4(in_p0_d3)
  bus_expand5/5(out4) -> snap_dds1/1(in_p0_d0)
  bus_expand5/6(out3) -> snap_dds1/2(in_p0_d1)
  bus_expand5/7(out2) -> snap_dds1/3(in_p0_d2)
  bus_expand5/8(lsb_out1) -> snap_dds1/4(in_p0_d3)
  Gateway Out34/1 -> To Workspace34/1
  Constant17/1 -> White Gaussian Noise/1
  Constant19/1 -> White Gaussian Noise/2
  White Gaussian Noise/1 -> Reinterpret/1
  Reinterpret/1 -> Slice/1
  pipeline14/1(q) -> Mux/2, AddSub2/1
  Constant30/1 -> gap_sel/1(sim_1)
  gap_sel/1(in_sel) -> pipeline8/1(d)
  pipeline8/1(q) -> Mux/1
  Mux/1 -> Register/1
  Register/1 -> AddSub3/1, Gateway Out38/1
  Gateway Out37/1 -> To Workspace37/1
  Gateway Out38/1 -> To Workspace38/1
  Gateway Out39/1 -> To Workspace39/1
  Reinterpret1/1 -> Slice1/1
  White Gaussian Noise/2 -> Reinterpret1/1
  [pkt_len] -> Relational2/2
  Constant32/1 -> pkt_len_sel/1(sim_1)
  pkt_len_sel/1(in_sel) -> pipeline19/1(d)
  pipeline19/1(q) -> Mux1/1
  Constant33/1 -> pkt_len/1(sim_1)
  pipeline28/1(q) -> AddSub1/1, Mux1/2
  Gateway Out40/1 -> To Workspace40/1
  Mux1/1 -> Gateway Out40/1, [pkt_len]
  AddSub1/1 -> Mux1/3
  Slice1/1 -> AddSub1/2
  Delay1/1 -> edge_detect2/1(in)
  pkt_len/1(in_len) -> pipeline28/1(d)
  Slice/1 -> AddSub2/2
  AddSub2/1 -> Mux/3
  cwg2/3(dv_out) -> Delay6/1
  cwg2/2(data_out) -> Delay7/1
  Delay6/1 -> [cwg_dv], Gateway Out31/1
  Delay7/1 -> [cwg80]
  Delay8/1 -> [osc_cos_ddc0]
  Delay9/1 -> [osc_sin_ddc0]
  Delay10/1 -> Register2/1, Register2/3, Delay2/1
  [cwg_cmplx] -> [ddc_pol0_in]
  [ddc_sync] -> nb_pfb1/1(sync)
  [ddc_pol0] -> nb_pfb1/2(pol0)
  [ddc_pol1] -> nb_pfb1/3(pol1)
  [ddc_dvalid] -> nb_pfb1/4(dvalid)
  [fft_shift] -> nb_pfb1/5(fft_shift)
  [osc_temp_dv] -> Delay10/1
  Constant38/1 -> [osc_temp_dv]
  Constant34/1 -> freq_cwg_osc/1(sim_1)
  [pfb_pol0_0] -> Gateway Out95/1, bus_expand7/1(bus_in)
  Gateway Out53/1 -> To Workspace51/1
  bus_expand7/2(lsb_out1) -> Gateway Out53/1
  Gateway Out52/1 -> To Workspace52/1
  bus_expand7/1(msb_out2) -> Gateway Out52/1
  Gateway Out51/1 -> To Workspace53/1
  [pfb_sync0] -> Gateway Out51/1
  [pfb_dv0] -> Gateway Out54/1
  Gateway Out54/1 -> To Workspace54/1
  wng0/1(bus_out) -> bus_expand10/1(bus_in), [wng]
  pipeline46/1(q) -> [wng_scale0]
  [wng_scale0] -> wng0/1(scale_16_14)
  man_rst_wng0/1(in_rst) -> Logical20/2
  Logical20/1 -> pipeline198/1(d)
  pipeline198/1(q) -> wng0/2(rst)
  scale_wng0/1(in_scale) -> Gateway Out55/1, pipeline46/1(d)
  Constant72/1 -> man_rst_wng0/1(sim_1)
  Constant73/1 -> scale_wng0/1(sim_1)
  Gateway Out55/1 -> To Workspace55/1
  bus_addsub/1(dout) -> bus_convert2/1(din)
  bus_convert2/1(dout) -> [cwg_awgn]
  [cwg80] -> bus_addsub/1(a)
  [wng] -> bus_addsub/2(b)
  bus_addsub/2(misco) -> bus_convert2/2(misci)
  cwg2/1(sync_out) -> Gateway Out30/1, [cwg_sync]
  Concat1/1 -> bus_addsub/3(misci)
  [cwg_sync] -> Concat1/1
  [cwg_dv] -> Concat1/2
  bus_expand8/1(msb_out2) -> Gateway Out117/1, [ddc_sync_in]
  bus_expand8/2(lsb_out1) -> Gateway Out118/1, [ddc_dv_in]
  bus_convert2/2(misco) -> bus_expand8/1(bus_in)
  [sys_rst] -> Logical20/1
  nb_pfb1/1(sync_out) -> Delay144/1
  nb_pfb1/3(pol0_out) -> Delay142/1
  Delay143/1 -> Delay146/1
  Delay145/1 -> [pfb_sync0]
  Delay144/1 -> Delay145/1
  nb_pfb1/2(dv_out) -> Delay143/1
  Delay146/1 -> [pfb_dv0]
  nb_pfb1/6(pfb_ch_out) -> Convert7/1
  Gateway Out95/1 -> To Workspace95/1
  Delay142/1 -> Delay38/1
  Delay38/1 -> [pfb_pol0_0]
  Convert7/1 -> Delay40/1
  Delay40/1 -> [pfb_ch_out_0]
  Gateway Out35/1 -> To Workspace35/1
  [pfb_ch_out_0] -> Gateway Out35/1
  Gateway Out117/1 -> To Workspace117/1
  Gateway Out118/1 -> To Workspace118/1
  Delay17/1 -> [fft_ch_out_0]
  Convert1/1 -> Delay17/1
  Delay16/1 -> [fft_pol0_0]
  Delay11/1 -> Delay16/1
  nb_pfb2/6(fft_ch_out) -> Convert1/1
  Delay15/1 -> [fft_dv0]
  nb_pfb2/2(dv_out) -> Delay12/1
  Delay13/1 -> Delay14/1
  Delay14/1 -> [fft_sync0]
  Delay12/1 -> Delay15/1
  nb_pfb2/1(sync_out) -> Delay13/1
  [fft_shift] -> nb_both_pol/5(fft_shift), nb_pfb2/5(fft_shift)
  [ddc_dvalid] -> nb_both_pol/4(dvalid), nb_pfb2/4(dvalid)
  [ddc_pol1] -> nb_both_pol/3(pol1), nb_pfb2/3(pol1)
  [ddc_pol0] -> nb_both_pol/2(pol0), nb_pfb2/2(pol0)
  [ddc_sync] -> nb_pfb2/1(sync), nb_both_pol/1(sync)
  [fft_ch_out_0] -> Gateway Out36/1
  Gateway Out36/1 -> To Workspace36/1
  Gateway Out45/1 -> To Workspace45/1
  Gateway Out44/1 -> To Workspace44/1
  [fft_dv0] -> Gateway Out44/1
  [fft_sync0] -> Gateway Out41/1
  Gateway Out41/1 -> To Workspace43/1
  bus_expand6/1(msb_out2) -> Gateway Out42/1
  Gateway Out42/1 -> To Workspace42/1
  bus_expand6/2(lsb_out1) -> Gateway Out43/1
  Gateway Out43/1 -> To Workspace41/1
  [fft_pol0_0] -> bus_expand6/1(bus_in), bus_expand9/1(bus_in), Gateway Out45/1
  Constant8/1 -> freq_cwg_input/1(sim_1)
  Constant35/1 -> nb_pfb2/6(Enable_Native_FFT_outputs)
  nb_pfb2/3(pol0_out) -> Delay11/1
  Gateway Out47/1 -> To Workspace46/1
  Gateway Out46/1 -> To Workspace47/1
  bus_expand9/1(msb_out2) -> Convert2/1
  bus_expand9/2(lsb_out1) -> Convert3/1
  Convert2/1 -> Gateway Out46/1
  Convert3/1 -> Gateway Out47/1
  bus_expand10/1(msb_out8) -> Gateway Out48/1
  Gateway Out48/1 -> To Workspace48/1
  Gateway Out49/1 -> To Workspace49/1
  bus_expand10/3(out6) -> Gateway Out49/1
  Constant28/1 -> fft_shift/1(sim_1)
